simulink model "ofdm_txrx_supermimo_coded"  (Simulink 7.7)
settings: Solver=VariableStepDiscrete  SolverMode=SingleTasking  StartTime=0.0  StopTime=2*simOnly_simLength  RelTol=1e-3
PostLoadFcn:
  | ofdm_rx_supermimo_init
InitFcn:
  | ofdm_rx_supermimo_init
StartFcn:
  | ofdm_rx_supermimo_init
values from neighbouring files (symbols this model uses):
  ActionID_AFTransmit = 31    [ofdm_tx_supermimo_init.m]
  ActionID_Disabled = 0    [ofdm_tx_supermimo_init.m]
  ActionID_SetFlagA = 62    [ofdm_tx_supermimo_init.m]
  ActionID_SetFlagB = 61    [ofdm_tx_supermimo_init.m]
  BER_RAM_init_size = 2048    [ofdm_tx_supermimo_init.m]
  channel_h = [1 0; 1 0] * 0.3    [ofdm_tx_supermimo_init.m]
  CPLength = 16    [ofdm_tx_supermimo_init.m]
  longCorr_coef_i = [-4*real(LTS) 0]    [ofdm_rx_supermimo_init.m]
  longCorr_coef_q = [4*imag(LTS) 0]    [ofdm_rx_supermimo_init.m]
  max_CP_length = 16    [ofdm_tx_supermimo_init.m]
  max_num_baseRateSymbols = 31    [ofdm_tx_supermimo_init.m]
  max_num_subcarriers = 64    [ofdm_tx_supermimo_init.m]
  max_num_trainingSymbols = 15    [ofdm_tx_supermimo_init.m]
  max_numBytes = 16384    [ofdm_tx_supermimo_init.m]
  max_OFDM_symbols = 1023    [ofdm_tx_supermimo_init.m]
  modConstellation_qam16 = .75*[1 3 -1 -3]./3    [ofdm_tx_supermimo_init.m]
  modConstellation_qam64 = 0.875*[3 1 5 7 -3 -1 -5 -7]./7    [ofdm_tx_supermimo_init.m]
  modConstellation_qpsk = [1 -1]./sqrt(2)    [ofdm_tx_supermimo_init.m]
  numBaseRateSymbols = numBaseRateSymbols *2    [ofdm_tx_supermimo_init.m]
  numSubcarriers = 64    [ofdm_tx_supermimo_init.m]
  numTrainingSymbols = 2    [ofdm_tx_supermimo_init.m]
  pktTiming_count_payloadStart = pktTiming_count_lsStart + pktTiming_ls_num*pktTiming_ls_length    [ofdm_rx_supermimo_init.m]
  preamble = preamble_scale*[shortsyms_10 longsyms_2]    [ofdm_tx_supermimo_init.m]
  preamble_ROM = preamble_I_ROM + preamble_Q_ROM*2^16    [ofdm_tx_supermimo_init.m]
  RAM_init_size = max_numBytes    (= 16384)    [ofdm_tx_supermimo_init.m]
  RxDataScrambling_Seq = [40 198 78 63 82 173 102 245 48 111 172 115 147 230 216 93 72 65 62 2 205 242 122 90 128 83 105 97 73 10 5 252]    [ofdm_tx_supermimo_init.m]
  shortSymbol_time = shortSymbol_time(1:16).'    [ofdm_tx_supermimo_init.m]
  simOnly_numSamples = length(preamble)+( (numSubcarriers+CPLength)*(numTrainingSymbols + numBaseRateSymbols + numFullRateSymbols/2) )    [ofdm_tx_supermimo_init.m]
  train = [train train]    [ofdm_tx_supermimo_init.m]
  TxDataScrambling_Seq = [40 198 78 63 82 173 102 245 48 111 172 115 147 230 216 93 72 65 62 2 205 242 122 90 128 83 105 97 73 10 5 252]    [ofdm_tx_supermimo_init.m]
undefined symbols (not defined in the file or in its folder): AntA, AntB, BaseRate, Down, gcbh, numBuffs, numBytes, Up, xlBlockLoadCallback, xlGetNormalizedPeriod, xlipgetpartsetting
libraries: xbsIndex_r4 x5430
(part 5 of 49)

== system ofdm_txrx_supermimo_coded/Memory-mapped Registers/TxRx Registers ==
  16LSB+8 : xbsIndex_r4/Slice  nbits=16  boolean_output=off  mode=Lower Bit Location + Width  bit1=0  base1=MSB of Input  bit0=8  base0=LSB of Input  dbl_ovrd=off  block_type=slice
      settings: infoedit="Extracts a given range of bits from each input sample and presents it at the output.  The output type is ordinarily unsigned with binary point at zero, but can be Boolean when the slice is one bit wide.<P><P>Hardware notes: In hardware this block costs nothing."  block_version=VER_STRING_GOES_HERE
  16LSB1 : xbsIndex_r4/Slice  nbits=ceil(log2(max_num_trainingSymbols))  boolean_output=off  mode=Lower Bit Location + Width  bit1=0  base1=MSB of Input  bit0=0  base0=LSB of Input  dbl_ovrd=off  block_type=slice
      settings: infoedit="Extracts a given range of bits from each input sample and presents it at the output.  The output type is ordinarily unsigned with binary point at zero, but can be Boolean when the slice is one bit wide.<P><P>Hardware notes: In hardware this block costs nothing."  block_version=VER_STRING_GOES_HERE
  16MSB1 : xbsIndex_r4/Slice  nbits=ceil(log2(max_num_baseRateSymbols))  boolean_output=off  mode=Lower Bit Location + Width  bit1=0  base1=MSB of Input  bit0=16  base0=LSB of Input  dbl_ovrd=off  block_type=slice
      settings: infoedit="Extracts a given range of bits from each input sample and presents it at the output.  The output type is ordinarily unsigned with binary point at zero, but can be Boolean when the slice is one bit wide.<P><P>Hardware notes: In hardware this block costs nothing."  block_version=VER_STRING_GOES_HERE
  6LSB+6 : xbsIndex_r4/Slice  nbits=6  boolean_output=off  mode=Lower Bit Location + Width  bit1=0  base1=MSB of Input  bit0=6  base0=LSB of Input  dbl_ovrd=off  block_type=slice
      settings: infoedit="Extracts a given range of bits from each input sample and presents it at the output.  The output type is ordinarily unsigned with binary point at zero, but can be Boolean when the slice is one bit wide.<P><P>Hardware notes: In hardware this block costs nothing."  block_version=VER_STRING_GOES_HERE
  6LSB1 : xbsIndex_r4/Slice  nbits=6  boolean_output=off  mode=Lower Bit Location + Width  bit1=0  base1=MSB of Input  bit0=0  base0=LSB of Input  dbl_ovrd=off  block_type=slice
      settings: infoedit="Extracts a given range of bits from each input sample and presents it at the output.  The output type is ordinarily unsigned with binary point at zero, but can be Boolean when the slice is one bit wide.<P><P>Hardware notes: In hardware this block costs nothing."  block_version=VER_STRING_GOES_HERE
  8LSB : xbsIndex_r4/Slice  nbits=8  boolean_output=off  mode=Lower Bit Location + Width  bit1=0  base1=MSB of Input  bit0=0  base0=LSB of Input  dbl_ovrd=off  block_type=slice
      settings: infoedit="Extracts a given range of bits from each input sample and presents it at the output.  The output type is ordinarily unsigned with binary point at zero, but can be Boolean when the slice is one bit wide.<P><P>Hardware notes: In hardware this block costs nothing."  block_version=VER_STRING_GOES_HERE
  AddSub1 : xbsIndex_r4/AddSub  mode=Addition  use_carryin=off  use_carryout=off  en=off  latency=0  precision=User Defined  arith_type=Unsigned  n_bits=1 + ceil(log2(max_num_trainingSymbols + max_num_baseRateSymbols))  bin_pt=0  quantization=Truncate  overflow=Wrap  dbl_ovrd=off  use_behavioral_HDL=on  hw_selection=Fabric  pipelined=off  use_rpm=on  block_type=addsub
      settings: xl_area=[0, 0, 0, 0, 0, 0, 0]  block_version=VER_STRING_GOES_HERE
  Down Sample{1,4,7,11,25} (5 alike) : xbsIndex_r4/Down Sample  sample_ratio=2  sample_phase="Last Value of Frame  (most efficient)"  en=off  latency=1  dbl_ovrd=off  block_type=dsamp
      settings: block_version=VER_STRING_GOES_HERE
  From Register2 : xbsIndex_r4/From Register  shared_memory_name='TxRx_FFT_Scaling'  init=TxRx_FFTScaling  period=1  ownership=Locally owned and initialized  gui_display_data_type=Fixed-point  arith_type=Unsigned  n_bits=12  bin_pt=0  preci_type=Single  dbl_ovrd=off  block_type=fromreg
      settings: AttributesFormatString=<< %<shared_memory_name> >>  infoedit="Register block that reads data to a shared memory register.  Delay of one sample period."
  From Register3 : xbsIndex_r4/From Register  shared_memory_name='TxRx_Interrupt_PktBuf_Ctrl'  init=reg_Interrupt_PktBuf_Control  period=1  ownership=Locally owned and initialized  gui_display_data_type=Fixed-point  arith_type=Unsigned  n_bits=32  bin_pt=0  preci_type=Single  dbl_ovrd=off  block_type=fromreg
      settings: AttributesFormatString=<< %<shared_memory_name> >>  infoedit="Register block that reads data to a shared memory register.  Delay of one sample period."
  From Register4 : xbsIndex_r4/From Register  shared_memory_name='TxRx_Pilots_Index'  init=pilot_indicies  period=1  ownership=Locally owned and initialized  gui_display_data_type=Fixed-point  arith_type=Unsigned  n_bits=32  bin_pt=0  preci_type=Single  dbl_ovrd=off  block_type=fromreg
      settings: AttributesFormatString=<< %<shared_memory_name> >>  infoedit="Register block that reads data to a shared memory register.  Delay of one sample period."
  From Register5 : xbsIndex_r4/From Register  shared_memory_name='TxRx_Pilots_Values'  init=pilotValues  period=1  ownership=Locally owned and initialized  gui_display_data_type=Fixed-point  arith_type=Unsigned  n_bits=32  bin_pt=0  preci_type=Single  dbl_ovrd=off  block_type=fromreg
      settings: AttributesFormatString=<< %<shared_memory_name> >>  infoedit="Register block that reads data to a shared memory register.  Delay of one sample period."
  From Register6 : xbsIndex_r4/From Register  shared_memory_name='Rx_OFDM_SymbolCounts'  init=(2^16 * numBaseRateSymbols) + numTrainingSymbols  period=1  ownership=Locally owned and initialized  gui_display_data_type=Fixed-point  arith_type=Unsigned  n_bits=32  bin_pt=0  preci_type=Single  dbl_ovrd=off  block_type=fromreg
      settings: AttributesFormatString=<< %<shared_memory_name> >>  infoedit="Register block that reads data to a shared memory register.  Delay of one sample period."
  From Register7 : xbsIndex_r4/From Register  shared_memory_name='Tx_OFDM_SymCounts'  init=txReg_symbolCounts  period=1  ownership=Locally owned and initialized  gui_display_data_type=Fixed-point  arith_type=Unsigned  n_bits=32  bin_pt=0  preci_type=Single  dbl_ovrd=off  block_type=fromreg
      settings: AttributesFormatString=<< %<shared_memory_name> >>  infoedit="Register block that reads data to a shared memory register.  Delay of one sample period."
  From Register8 : xbsIndex_r4/From Register  shared_memory_name='FEC_Config'  init=FEC_Config  period=1  ownership=Locally owned and initialized  gui_display_data_type=Fixed-point  arith_type=Unsigned  n_bits=32  bin_pt=0  preci_type=Single  dbl_ovrd=off  block_type=fromreg
      settings: AttributesFormatString=<< %<shared_memory_name> >>  infoedit="Register block that reads data to a shared memory register.  Delay of one sample period."
  InterruptControl : Subsystem
  LSB : xbsIndex_r4/Slice  nbits=1  boolean_output=off  mode=Lower Bit Location + Width  bit1=0  base1=MSB of Input  bit0=0  base0=LSB of Input  dbl_ovrd=off  block_type=slice
      settings: infoedit="Extracts a given range of bits from each input sample and presents it at the output.  The output type is ordinarily unsigned with binary point at zero, but can be Boolean when the slice is one bit wide.<P><P>Hardware notes: In hardware this block costs nothing."  block_version=VER_STRING_GOES_HERE
  tag scope: Coding_En=global, FEC_Reg=global, Rx_FFTScaling=global, TxReg_OFDM_SymCounts=global, Tx_FFTScaling=global, regRx_numBaseRateSymbols=global, regRx_numTrainPlusBaseRateSyms=global, regRx_numTrainingSymbols=global, regTxRx_PilotToneIndex=global, regTxRx_PktBuffOffsets=global, regTx_PilotToneValues=global
  wires:
  From Register3/1 -> Down Sample7/1, 16LSB+8/1
  Down Sample7/1 -> 8LSB/1
  From Register2/1 -> Down Sample25/1
  Down Sample25/1 -> 6LSB1/1, 6LSB+6/1
  6LSB1/1 -> [Tx_FFTScaling]
  6LSB+6/1 -> [Rx_FFTScaling]
  16LSB+8/1 -> [regTxRx_PktBuffOffsets]
  8LSB/1 -> InterruptControl/1(Reg_8b)
  From Register4/1 -> Down Sample4/1
  Down Sample4/1 -> [regTxRx_PilotToneIndex]
  From Register5/1 -> [regTx_PilotToneValues]
  From Register7/1 -> [TxReg_OFDM_SymCounts]
  AddSub1/1 -> [regRx_numTrainPlusBaseRateSyms]
  16LSB1/1 -> [regRx_numTrainingSymbols], AddSub1/1
  16MSB1/1 -> [regRx_numBaseRateSymbols], AddSub1/2
  From Register6/1 -> Down Sample11/1
  Down Sample11/1 -> 16MSB1/1, 16LSB1/1
  From Register8/1 -> LSB/1, [FEC_Reg]
  LSB/1 -> Down Sample1/1
  Down Sample1/1 -> [Coding_En]

== system ofdm_txrx_supermimo_coded/OFDM Rx MIMO/ADC Inputs & Antenna Selection ==
  note: Extra latency to cover delay through
      | MAX2829 amps -> ADC -> FPGA
  PktDetReset : Inport
  "A I " : Inport  Port=2
  "A Q " : Inport  Port=3
  "B I " : Inport  Port=4
  "B Q " : Inport  Port=5
  Antenna Selection : Subsystem
  Constant4 : Constant
  Constant5 : Constant
  Convert : xbsIndex_r4/Convert  gui_display_data_type=Fixed-point  arith_type=Boolean  n_bits=16  bin_pt=14  float_type=Single  exp_bits=8  fraction_bits=24  quantization=Truncate  overflow=Wrap  en=off  latency=0  dbl_ovrd=off  pipeline=off  block_type=convert
      settings: block_version=10.1.3
  Convert1 : xbsIndex_r4/Convert  gui_display_data_type=Fixed-point  arith_type=Boolean  n_bits=16  bin_pt=14  float_type=Single  exp_bits=8  fraction_bits=24  quantization=Truncate  overflow=Wrap  en=off  latency=0  dbl_ovrd=off  pipeline=off  block_type=convert
      settings: block_version=10.1.3
  Down Sample{,1..3} (4 alike) : xbsIndex_r4/Down Sample  sample_ratio=4  sample_phase="Last Value of Frame  (most efficient)"  en=off  latency=1  dbl_ovrd=off  block_type=dsamp
      settings: block_version=8.2.02
  Down Sample6 : xbsIndex_r4/Down Sample  sample_ratio=4  sample_phase="Last Value of Frame  (most efficient)"  en=off  latency=4  dbl_ovrd=off  block_type=dsamp
      settings: block_version=8.2.02
  Down Sample9 : xbsIndex_r4/Down Sample  sample_ratio=4  sample_phase="Last Value of Frame  (most efficient)"  en=off  latency=4  dbl_ovrd=off  block_type=dsamp
      settings: block_version=8.2.02
  Inverter : xbsIndex_r4/Inverter  en=off  latency=0  dbl_ovrd=off  block_type=inv
  Inverter1 : xbsIndex_r4/Inverter  en=off  latency=0  dbl_ovrd=off  block_type=inv
  Inverter2 : xbsIndex_r4/Inverter  en=off  latency=0  dbl_ovrd=off  block_type=inv
  Logical1 : xbsIndex_r4/Logical  logical_function=AND  inputs=2  en=off  latency=0  precision=Full  arith_type=Unsigned  n_bits=8  bin_pt=2  align_bp=on  dbl_ovrd=off  block_type=logical
      settings: xl_area=[1 0 0 1 0 0 0]  block_version=VER_STRING_GOES_HERE
  Logical2 : xbsIndex_r4/Logical  logical_function=OR  inputs=2  en=off  latency=0  precision=Full  arith_type=Unsigned  n_bits=8  bin_pt=2  align_bp=on  dbl_ovrd=off  block_type=logical
      settings: xl_area=[1 0 0 1 0 0 0]  block_version=VER_STRING_GOES_HERE
  Logical3 : xbsIndex_r4/Logical  logical_function=AND  inputs=2  en=off  latency=0  precision=Full  arith_type=Unsigned  n_bits=8  bin_pt=2  align_bp=on  dbl_ovrd=off  block_type=logical
      settings: xl_area=[1 0 0 1 0 0 0]  block_version=VER_STRING_GOES_HERE
  Register{,1..3} (4 alike) : xbsIndex_r4/Register  init=0  rst=on  en=off  dbl_ovrd=off  block_type=register
  Rx_AntA_ADCI : xbsIndex_r4/Gateway In  gui_display_data_type=Fixed-point  arith_type="Signed  (2's comp)"  n_bits=14  bin_pt=13  preci_type=Single  exp_width=8  frac_width=24  quantization="Round  (unbiased: +/- Inf)"  overflow=Saturate  period=1  dbl_ovrd=off  timing_constraint=None  locs_specified=off  LOCs={}  inherit_from_input=off  UseAsADC=off  ADCChannel='1'  hdl_port=on  block_type=gatewayin
      settings: infoedit="Gateway in block.  Converts inputs of type Simulink integer, double and fixed point to  Xilinx fixed point type.<P><P>Hardware notes:  In hardware these blocks become top level input ports."  block_version=VER_STRING_GOES_HERE
  Rx_AntA_ADCQ : xbsIndex_r4/Gateway In  gui_display_data_type=Fixed-point  arith_type="Signed  (2's comp)"  n_bits=14  bin_pt=13  preci_type=Single  exp_width=8  frac_width=24  quantization="Round  (unbiased: +/- Inf)"  overflow=Saturate  period=1  dbl_ovrd=off  timing_constraint=None  locs_specified=off  LOCs={}  inherit_from_input=off  UseAsADC=off  ADCChannel='1'  hdl_port=on  block_type=gatewayin
      settings: infoedit="Gateway in block.  Converts inputs of type Simulink integer, double and fixed point to  Xilinx fixed point type.<P><P>Hardware notes:  In hardware these blocks become top level input ports."  block_version=VER_STRING_GOES_HERE
  Rx_AntA_AGC_Done : xbsIndex_r4/Gateway In  gui_display_data_type=Fixed-point  arith_type=Unsigned  n_bits=1  bin_pt=0  preci_type=Single  exp_width=8  frac_width=24  quantization="Round  (unbiased: +/- Inf)"  overflow=Saturate  period=1  dbl_ovrd=off  timing_constraint=None  locs_specified=off  LOCs={}  inherit_from_input=off  UseAsADC=off  ADCChannel='1'  hdl_port=on  block_type=gatewayin
      settings: infoedit="Gateway in block.  Converts inputs of type Simulink integer, double and fixed point to  Xilinx fixed point type.<P><P>Hardware notes:  In hardware these blocks become top level input ports."  block_version=VER_STRING_GOES_HERE
  Rx_AntB_ADCI : xbsIndex_r4/Gateway In  gui_display_data_type=Fixed-point  arith_type="Signed  (2's comp)"  n_bits=14  bin_pt=13  preci_type=Single  exp_width=8  frac_width=24  quantization="Round  (unbiased: +/- Inf)"  overflow=Saturate  period=1  dbl_ovrd=off  timing_constraint=None  locs_specified=off  LOCs={}  inherit_from_input=off  UseAsADC=off  ADCChannel='1'  hdl_port=on  block_type=gatewayin
      settings: infoedit="Gateway in block.  Converts inputs of type Simulink integer, double and fixed point to  Xilinx fixed point type.<P><P>Hardware notes:  In hardware these blocks become top level input ports."  block_version=VER_STRING_GOES_HERE
  Rx_AntB_ADCQ : xbsIndex_r4/Gateway In  gui_display_data_type=Fixed-point  arith_type="Signed  (2's comp)"  n_bits=14  bin_pt=13  preci_type=Single  exp_width=8  frac_width=24  quantization="Round  (unbiased: +/- Inf)"  overflow=Saturate  period=1  dbl_ovrd=off  timing_constraint=None  locs_specified=off  LOCs={}  inherit_from_input=off  UseAsADC=off  ADCChannel='1'  hdl_port=on  block_type=gatewayin
      settings: infoedit="Gateway in block.  Converts inputs of type Simulink integer, double and fixed point to  Xilinx fixed point type.<P><P>Hardware notes:  In hardware these blocks become top level input ports."  block_version=VER_STRING_GOES_HERE
  Rx_AntB_AGC_Done : xbsIndex_r4/Gateway In  gui_display_data_type=Fixed-point  arith_type=Unsigned  n_bits=1  bin_pt=0  preci_type=Single  exp_width=8  frac_width=24  quantization="Round  (unbiased: +/- Inf)"  overflow=Saturate  period=1  dbl_ovrd=off  timing_constraint=None  locs_specified=off  LOCs={}  inherit_from_input=off  UseAsADC=off  ADCChannel='1'  hdl_port=on  block_type=gatewayin
      settings: infoedit="Gateway in block.  Converts inputs of type Simulink integer, double and fixed point to  Xilinx fixed point type.<P><P>Hardware notes:  In hardware these blocks become top level input ports."  block_version=VER_STRING_GOES_HERE
  A I : Outport
  A Q : Outport  Port=2
  B I : Outport  Port=3
  B Q : Outport  Port=4
  tag scope: AntA_ADC_I_Filt=global, AntA_ADC_Q_Filt=global, AntA_AGC_Done=global, AntB_AGC_Done=global
  wires:
  "B Q "/1 -> Rx_AntB_ADCQ/1
  "B I "/1 -> Rx_AntB_ADCI/1
  "A Q "/1 -> Rx_AntA_ADCQ/1
  "A I "/1 -> Rx_AntA_ADCI/1
  Down Sample/1 -> Antenna Selection/1(AntA_I)
  Down Sample1/1 -> Antenna Selection/2(AntA_Q)
  Down Sample2/1 -> Antenna Selection/3(AntB_I)
  Down Sample3/1 -> Antenna Selection/4(AntB_Q)
  Antenna Selection/1(A I) -> [AntA_ADC_I_Filt], Register/1
  Antenna Selection/2(A Q) -> [AntA_ADC_Q_Filt], Register1/1
  PktDetReset/1 -> Antenna Selection/5(PktDetReset)
  Constant5/1 -> Rx_AntB_AGC_Done/1
  Rx_AntB_AGC_Done/1 -> Down Sample9/1
  Down Sample9/1 -> [AntB_AGC_Done], Convert1/1
  Constant4/1 -> Rx_AntA_AGC_Done/1
  Rx_AntA_AGC_Done/1 -> Down Sample6/1
  Down Sample6/1 -> [AntA_AGC_Done], Convert/1
  Rx_AntA_ADCI/1 -> Down Sample/1
  Rx_AntA_ADCQ/1 -> Down Sample1/1
  Rx_AntB_ADCI/1 -> Down Sample2/1
  Rx_AntB_ADCQ/1 -> Down Sample3/1
  Register/1 -> A I/1
  Register1/1 -> A Q/1
  Register2/1 -> B I/1
  Register3/1 -> B Q/1
  Antenna Selection/4(B Q) -> Register3/1
  Antenna Selection/3(B I) -> Register2/1
  Inverter1/1 -> Register2/2, Register3/2, Logical3/2
  Convert/1 -> Inverter/1
  Convert1/1 -> Inverter1/1
  Inverter/1 -> Logical1/2
  Inverter2/1 -> Logical1/1
  Antenna Selection/5(A_swapped) -> Inverter2/1, Logical3/1
  Logical1/1 -> Logical2/1
  Logical3/1 -> Logical2/2
  Logical2/1 -> Register1/2, Register/2

== system ofdm_txrx_supermimo_coded/OFDM Rx MIMO/AF Buffers ==
  PktDone : Inport
  Payload : Inport  Port=2
  AntA_I : Inport  Port=3
  AntA_Q : Inport  Port=4
  AF Buffers : Subsystem
  tag scope: AF_OutputSamples_I=global, AF_OutputSamples_Q=global, AF_PreambleIndex=global, AF_TxDone=global
  wires:
  AntA_I/1 -> AF Buffers/1(AntA_I)
  AntA_Q/1 -> AF Buffers/2(AntA_Q)
  Payload/1 -> AF Buffers/3(Payload)
  PktDone/1 -> AF Buffers/4(PktDone)
  AF Buffers/1(I) -> [AF_OutputSamples_I]
  AF Buffers/2(Q) -> [AF_OutputSamples_Q]
  AF Buffers/3(AF Pream Index) -> [AF_PreambleIndex]
  AF Buffers/4(AFTx Done) -> [AF_TxDone]

== system ofdm_txrx_supermimo_coded/OFDM Rx MIMO/Coarse Freq Correction ==
  note: Potential for overflow pre-AGC
      | (but not post? I think it's ok)
      | |
      | V
  Reset : Inport
  PktDet : Inport  Port=2
  AntA I : Inport  Port=3
  AntA Q : Inport  Port=4
  AntB I : Inport  Port=5
  AntB Q : Inport  Port=6
  A*B : Subsystem
  A*B1 : Subsystem
  Bus Creator1 : BusCreator  Inputs=2
  CFO : Scope  NumInputPorts=5
      settings: TimeRange=14608  YMin=0.000246735~0.95~-0.3~-1~-1  YMax=0.000272707~1.05~1~1~1  SaveName=ScopeData26
  Coarse CFO Calc : Subsystem
  Complex to Magnitude-Angle : ComplexToMagnitudeAngle
  Complex to Real-Imag : ComplexToRealImag
  Convert{,1..3} (4 alike) : xbsIndex_r4/Convert  gui_display_data_type=Fixed-point  arith_type="Signed  (2's comp)"  n_bits=16  bin_pt=15  float_type=Single  exp_bits=8  fraction_bits=24  quantization=Truncate  overflow=Wrap  en=off  latency=0  dbl_ovrd=off  pipeline=off  block_type=convert
      settings: xl_area=[0 0 0 0 0 0 0]  block_version=VER_STRING_GOES_HERE
  DDS : Subsystem
  Gateway Out{,1..3} (4 alike) : xbsIndex_r4/Gateway Out  inherit_from_input=off  hdl_port=off  timing_constraint=None  locs_specified=off  LOCs={}  UseAsDAC=off  DACChannel='1'  block_type=gatewayout
      settings: infoedit="Gateway out block.  Converts Xilinx fixed point inputs into ouputs of type Simulink integer, double, or fixed point.<P><P>Hardware notes:  In hardware these blocks become top level output ports or are discarded, depending on how they are configured."  xl_area=[0 0 0 0 0 0 0]  block_version=VER_STRING_GOES_HERE
  Mux2 : Mux  Inputs=2
  PreSpin CFO Sel : Subsystem
  Product : Product  RndMeth=Zero
  Real-Imag to Complex : RealImagToComplex
  "AntA I " : Outport
  "AntA Q " : Outport  Port=2
  "AntB I " : Outport  Port=3
  "AntB Q " : Outport  Port=4
  tag scope: CFO_PhaseInc=global
  wires:
  A*B/1(Re) -> Convert/1
  A*B/2(Im1) -> Convert1/1
  A*B1/1(Re) -> Convert2/1
  A*B1/2(Im1) -> Convert3/1
  DDS/1(Q) -> Gateway Out/1, A*B1/2(Im[B]), A*B/3(Im[B])
  DDS/2(I) -> Gateway Out1/1, A*B1/1(Re[B]), A*B/4(Re[B])
  Gateway Out1/1 -> Mux2/1, Real-Imag to Complex/1
  Gateway Out/1 -> Mux2/2, Real-Imag to Complex/2
  Mux2/1 -> CFO/3
  Bus Creator1/1 -> CFO/4
  [freqOffset] -> Complex to Real-Imag/1, Product/2
  Complex to Real-Imag/1 -> Bus Creator1/1
  Complex to Real-Imag/2 -> Bus Creator1/2
  Gateway Out2/1 -> CFO/2
  Gateway Out3/1 -> CFO/1
  AntB I/1 -> A*B1/3(Re[A])
  AntB Q/1 -> A*B1/4(Im[A])
  AntA I/1 -> A*B/1(Re[A]), Coarse CFO Calc/1(I In)
  AntA Q/1 -> A*B/2(Im[A]), Coarse CFO Calc/2(Q In)
  Convert/1 -> "AntA I "/1
  Convert1/1 -> "AntA Q "/1
  Real-Imag to Complex/1 -> Product/1
  Product/1 -> Complex to Magnitude-Angle/1
  Complex to Magnitude-Angle/2 -> CFO/5
  Convert2/1 -> "AntB I "/1
  Convert3/1 -> "AntB Q "/1
  Reset/1 -> Coarse CFO Calc/4(Reset), DDS/3(Rst), PreSpin CFO Sel/1(Rst)
  PktDet/1 -> Gateway Out2/1, Coarse CFO Calc/3(RxPkt Active)
  Coarse CFO Calc/1(Phase Inc) -> Gateway Out3/1, [CFO_PhaseInc], DDS/2(Rx_CoarseEst)
  PreSpin CFO Sel/1(Tx_PhaseInc) -> DDS/1(Tx_PhaseInc)

== system ofdm_txrx_supermimo_coded/OFDM Rx MIMO/Equalizer & Packetizer ==
  Payload : Inport
  Y_I : Inport  Port=2
  Y_Q : Inport  Port=3
  H-I : Inport  Port=4
  H-Q : Inport  Port=5
  SubCar Index : Inport  Port=6
  Sym Index : Inport  Port=7
  Vin : Inport  Port=8
  Phases I : Inport  Port=9
  Phases Q : Inport  Port=10
  Convert4 : xbsIndex_r4/Convert  gui_display_data_type=Fixed-point  arith_type="Signed  (2's comp)"  n_bits=14  bin_pt=13  float_type=Single  exp_bits=8  fraction_bits=24  quantization=Truncate  overflow=Wrap  en=off  latency=0  dbl_ovrd=off  pipeline=off  block_type=convert
      settings: xl_area=[0, 0, 0, 0, 0, 0, 0]  block_version=VER_STRING_GOES_HERE
  Convert5 : xbsIndex_r4/Convert  gui_display_data_type=Fixed-point  arith_type="Signed  (2's comp)"  n_bits=14  bin_pt=13  float_type=Single  exp_bits=8  fraction_bits=24  quantization=Truncate  overflow=Wrap  en=off  latency=0  dbl_ovrd=off  pipeline=off  block_type=convert
      settings: xl_area=[0, 0, 0, 0, 0, 0, 0]  block_version=VER_STRING_GOES_HERE
  Eq : Scope  NumInputPorts=7
      settings: YMin=-0.5~-0.5~0~0~0~0~-1  YMax=0.5~0.5~70~1~22.5~1~1  SaveName=ScopeData14
  Equalizer : Subsystem
  Packet_Constructor : Subsystem
  Real-Imag to Complex : RealImagToComplex
  Rx_debug_EQ_I : xbsIndex_r4/Gateway Out  inherit_from_input=off  hdl_port=on  timing_constraint=None  locs_specified=off  LOCs={}  UseAsDAC=off  DACChannel='1'  block_type=gatewayout
      settings: infoedit="Gateway out block.  Converts Xilinx fixed point inputs into ouputs of type Simulink integer, double, or fixed point.<P><P>Hardware notes:  In hardware these blocks become top level output ports or are discarded, depending on how they are configured."  xl_area=[0 0 0 0 1 0 0]  block_version=VER_STRING_GOES_HERE
  Rx_debug_EQ_Q : xbsIndex_r4/Gateway Out  inherit_from_input=off  hdl_port=on  timing_constraint=None  locs_specified=off  LOCs={}  UseAsDAC=off  DACChannel='1'  block_type=gatewayout
      settings: infoedit="Gateway out block.  Converts Xilinx fixed point inputs into ouputs of type Simulink integer, double, or fixed point.<P><P>Hardware notes:  In hardware these blocks become top level output ports or are discarded, depending on how they are configured."  xl_area=[0 0 0 0 1 0 0]  block_version=VER_STRING_GOES_HERE
  Scaling : Subsystem
  Up Sample1 : xbsIndex_r4/Up Sample  sample_ratio=2  copy_samples=on  en=off  latency=0  dbl_ovrd=off  block_type=usamp
      settings: infoedit="Up samples input data.  Inserted values  can be zeros or copies of the most recent input sample.<P><P>Hardware notes: No hardware is needed if inserted values are copies of the input sample; otherwise, a mux and single bit flip-flop are used."  block_version=8.2.02
  Up Sample2 : xbsIndex_r4/Up Sample  sample_ratio=2  copy_samples=on  en=off  latency=0  dbl_ovrd=off  block_type=usamp
      settings: infoedit="Up samples input data.  Inserted values  can be zeros or copies of the most recent input sample.<P><P>Hardware notes: No hardware is needed if inserted values are copies of the input sample; otherwise, a mux and single bit flip-flop are used."  block_version=8.2.02
  Zero-Order Hold : ZeroOrderHold
  Zero-Order Hold1 : ZeroOrderHold
  done{1..6} (6 alike) : xbsIndex_r4/Gateway Out  inherit_from_input=off  hdl_port=off  timing_constraint=None  locs_specified=off  LOCs={}  UseAsDAC=off  DACChannel='1'  block_type=gatewayout
      settings: infoedit="Gateway out block.  Converts Xilinx fixed point inputs into ouputs of type Simulink integer, double, or fixed point.<P><P>Hardware notes:  In hardware these blocks become top level output ports or are discarded, depending on how they are configured."  xl_area=[0 0 0 0 1 0 0]  block_version=VER_STRING_GOES_HERE
  posedge : Subsystem
  done : Outport
  wires:
  SubCar Index/1 -> Equalizer/9(SubCar Index)
  Sym Index/1 -> Equalizer/8(Sym Index)
  Scaling/1(I) -> Packet_Constructor/1(I), Convert4/1
  Scaling/2(Q) -> Packet_Constructor/2(Q), Convert5/1
  Scaling/3(" Xk_index") -> Packet_Constructor/3(Xk_index)
  Scaling/4(Vout) -> Packet_Constructor/4(Vin)
  Scaling/5(" Sym Index") -> Packet_Constructor/5(Sym Index)
  Packet_Constructor/1(done) -> done/1
  H-I/1 -> Equalizer/5(H-I)
  H-Q/1 -> Equalizer/6(H-Q)
  Vin/1 -> Equalizer/7(Vin)
  Y_I/1 -> Equalizer/3(Y_I)
  Y_Q/1 -> Equalizer/4(Y_Q)
  Payload/1 -> posedge/1(D), done6/1
  posedge/1(Q) -> Packet_Constructor/6(Start)
  Equalizer/1(I-eq) -> Scaling/1(Eq-I), done4/1
  Equalizer/2(Q-eq) -> Scaling/2(Eq-Q), done5/1
  Equalizer/3(" Xk_index") -> Scaling/3(Xk_index), done1/1
  Equalizer/4(Vout) -> Scaling/4(Vin), done2/1
  Equalizer/5(" Sym Index") -> Scaling/5(Sym Index), done3/1
  Up Sample2/1 -> Rx_debug_EQ_Q/1
  Up Sample1/1 -> Rx_debug_EQ_I/1
  Convert5/1 -> Up Sample2/1
  Convert4/1 -> Up Sample1/1
  Phases I/1 -> Equalizer/1(Phases I)
  Phases Q/1 -> Equalizer/2(Phases Q)
  Rx_debug_EQ_I/1 -> Zero-Order Hold/1
  Rx_debug_EQ_Q/1 -> Zero-Order Hold1/1
  Real-Imag to Complex/1 -> ?
  Zero-Order Hold/1 -> Real-Imag to Complex/1
  Zero-Order Hold1/1 -> Real-Imag to Complex/2
  done4/1 -> Eq/1
  done5/1 -> Eq/2
  done1/1 -> Eq/3
  done2/1 -> Eq/4
  done3/1 -> Eq/5
  done6/1 -> Eq/6

== system ofdm_txrx_supermimo_coded/OFDM Rx MIMO/FFT & Chan Est ==
  note: Payload->WE latency was 1 - why?
  Payload : Inport
  AntA I : Inport  Port=2
  AntA Q : Inport  Port=3
  AntB I : Inport  Port=4
  AntB Q : Inport  Port=5
  Addr Gen1 : Subsystem
  Addr_Generator : Subsystem
  Assert : xbsIndex_r4/Assert  assert_type=off  type_source=Explicitly  gui_display_data_type=Fixed-point  arith_type="Signed  (2's comp)"  n_bits=16  bin_pt=14  preci_type=Single  exp_width=8  frac_width=24  assert_rate=on  rate_source=Explicitly  period=1  output_port=on  block_type=assert
      settings: infoedit=Asserts a user-defined sample rate and/or type on a signal.<P><P>Hardware notes: In hardware this block costs nothing.  block_version=VER_STRING_GOES_HERE
  Channel Estimation & Pilot Processing : Subsystem
  Delay{1..4} (4 alike) : xbsIndex_r4/Delay  rst=off  infoeditControl=Selection of Reset will increase slice count due to use of real FFs and instead of SRLs  en=off  latency=1  dbl_ovrd=off  reg_retiming=off  block_type=delay
      settings: infoedit=Hardware notes: A delay line is a chain, each link of which is an SRL16 followed by a flip-flop. If register retiming is enabled, the delay line is a chain of flip-flops.  block_version=VER_STRING_GOES_HERE
  Down Sample : xbsIndex_r4/Down Sample  sample_ratio=2  sample_phase=First Value of Frame  en=off  latency=0  dbl_ovrd=off  block_type=dsamp
      settings: block_version=VER_STRING_GOES_HERE
  FFT : Subsystem
  Input Buffers : Subsystem
  Inverter2 : xbsIndex_r4/Inverter  en=off  latency=0  dbl_ovrd=off  block_type=inv
      settings: block_version=VER_STRING_GOES_HERE
  Output Buffers : Subsystem
  Symbol Counter : Subsystem
  Y_I : Outport
  Y_Q : Outport  Port=2
  H-I : Outport  Port=3
  H-Q : Outport  Port=4
  SubCar Index : Outport  Port=5
  Sym Index : Outport  Port=6
  Vout : Outport  Port=7
  Phase I : Outport  Port=8
  Phase Q : Outport  Port=9
  tag scope: FFT_I=global
  wires:
  Delay1/1 -> Input Buffers/5(We)
  FFT/5(Done) -> Symbol Counter/1(FFT Done), Addr Gen1/1(FFT Done)
  Addr_Generator/3(Ant Sel) -> Input Buffers/8(Ant Sel)
  Assert/1 -> Addr_Generator/2(FFT Xn_index)
  AntB Q/1 -> Input Buffers/4(AntB Q)
  AntB I/1 -> Input Buffers/3(AntB I)
  AntA Q/1 -> Input Buffers/2(AntA Q)
  AntA I/1 -> Input Buffers/1(AntA I)
  Payload/1 -> Delay1/1, Addr_Generator/1(Payload), Symbol Counter/2(Payload), Addr Gen1/2(Payload), Inverter2/1
  Addr_Generator/2(Read Addr) -> Input Buffers/7(Rd Addr)
  Addr_Generator/5(FFT Start) -> FFT/3(Start)
  Input Buffers/2(" Q") -> FFT/2(xn_Q)
  Input Buffers/1(" I") -> FFT/1(xn_I)
  Addr_Generator/1(Write Addr) -> Input Buffers/6(Wr Addr)
  Delay4/1 -> SubCar Index/1
  Delay2/1 -> Vout/1
  Output Buffers/2(" Q") -> Y_Q/1
  Channel Estimation & Pilot Processing/2(H-Q) -> H-Q/1
  Channel Estimation & Pilot Processing/1(H-I) -> H-I/1
  Output Buffers/1(" I") -> Y_I/1
  Channel Estimation & Pilot Processing/5(Est Vout) -> Down Sample/1
  Symbol Counter/1(Sym Index) -> Channel Estimation & Pilot Processing/5(Sym Index), Output Buffers/5(SymIndex)
  FFT/4(Vout) -> Channel Estimation & Pilot Processing/4(Vin), Output Buffers/4(FFT Vout)
  FFT/3(xk_Index) -> Channel Estimation & Pilot Processing/3(Xk Index), Output Buffers/3(Wr Addr)
  FFT/2(xk_Q) -> Output Buffers/2(Q), Channel Estimation & Pilot Processing/2(Q)
  FFT/1(xk_I) -> Channel Estimation & Pilot Processing/1(I), Output Buffers/1(I), [FFT_I]
  FFT/6(xn_Index) -> Assert/1
  Down Sample/1 -> Addr Gen1/3(NotTraining)
  Addr Gen1/2(Rd Addr) -> Output Buffers/6(Rd Addr), Channel Estimation & Pilot Processing/6(Est Index), Delay4/1
  Addr Gen1/3(Vout) -> Delay2/1
  Delay3/1 -> Sym Index/1
  Addr Gen1/1(SymIndex_out) -> Delay3/1
  Addr_Generator/4(Wr Slot) -> Input Buffers/9(Wr Slot)
  Channel Estimation & Pilot Processing/3(Phase I) -> Phase I/1
  Channel Estimation & Pilot Processing/4(Phase Q) -> Phase Q/1
  Inverter2/1 -> Channel Estimation & Pilot Processing/7(Rst)

== system ofdm_txrx_supermimo_coded/OFDM Rx MIMO/Long Corr HP ==
  note: This block was originally designed by Dr. Chris Dick (Cheif DSP Scientist at Xilinx)
      | It's been modified since to run at 4x (instead of 5x, as in the original OFDM designs)
      |
      | This block runs a 64-point 1-bit by 1-bit correlation per clock cycle. Using 1-bit per
      | operand allows just add/sub operations instead of actual multiplicaitons,
      | which would be hugely expensive. This degrades performance a little,
      | but the LTS have good enough autocorrelation properties that there is still
      | plenth of margin using this scheme.
  I In : Inport
  Q In : Inport  Port=2
  AddSub : xbsIndex_r4/AddSub  mode=Subtraction  use_carryin=off  use_carryout=off  en=off  latency=0  precision=Full  arith_type=Unsigned  n_bits=8  bin_pt=2  quantization=Truncate  overflow=Wrap  dbl_ovrd=off  use_behavioral_HDL=on  hw_selection=Fabric  pipelined=off  use_rpm=off  block_type=addsub
      settings: xl_area=[5 0 0 8 0 0 0]  block_version=VER_STRING_GOES_HERE
  AddSub1 : xbsIndex_r4/AddSub  mode=Addition  use_carryin=off  use_carryout=off  en=off  latency=0  precision=Full  arith_type=Unsigned  n_bits=8  bin_pt=2  quantization=Truncate  overflow=Wrap  dbl_ovrd=off  use_behavioral_HDL=on  hw_selection=Fabric  pipelined=off  use_rpm=off  block_type=addsub
      settings: xl_area=[5 0 0 8 0 0 0]  block_version=VER_STRING_GOES_HERE
  AddSub2 : xbsIndex_r4/AddSub  mode=Addition  use_carryin=off  use_carryout=off  en=off  latency=0  precision=Full  arith_type=Unsigned  n_bits=8  bin_pt=2  quantization=Truncate  overflow=Wrap  dbl_ovrd=off  use_behavioral_HDL=on  hw_selection=Fabric  pipelined=off  use_rpm=off  block_type=addsub
      settings: xl_area=[10 0 0 18 0 0 0]  block_version=VER_STRING_GOES_HERE
  C1 : Subsystem  mask{ns=0, N=4, h=longCorr_coef_i, Ts_In=4}
      mask prompts: ns "Num sub-correlator"; N "Coefficients/correlator"; h "Coefficients"; Ts_In "Input sample period"
  C2 : Subsystem  mask{ns=0, N=4, h=longCorr_coef_q, Ts_In=4}
      mask prompts: ns "Num sub-correlator"; N "Coefficients/correlator"; h "Coefficients"; Ts_In "Input sample period"
  C3 : Subsystem  mask{ns=0, N=4, h=longCorr_coef_i, Ts_In=4}
      mask prompts: ns "Num sub-correlator"; N "Coefficients/correlator"; h "Coefficients"; Ts_In "Input sample period"
  C4 : Subsystem  mask{ns=0, N=4, h=longCorr_coef_q, Ts_In=4}
      mask prompts: ns "Num sub-correlator"; N "Coefficients/correlator"; h "Coefficients"; Ts_In "Input sample period"
  Mult : xbsIndex_r4/Mult  precision=Full  arith_type="Signed  (2's comp)"  n_bits=8  bin_pt=2  quantization=Truncate  overflow=Wrap  en=off  latency=1  dbl_ovrd=off  use_behavioral_HDL=off  opt=Speed  use_embedded=on  optimum_pipeline=off  pipeline=on  use_rpm=off  placement_style=Rectangular shape  block_type=mult
      settings: infoedit=Hardware notes: To use the internal pipeline stage of the dedicated multiplier you must select 'Pipeline for maximum performance'.  xl_area=[60 18 0 106 0 0 0]  block_version=VER_STRING_GOES_HERE
  Mult1 : xbsIndex_r4/Mult  precision=Full  arith_type="Signed  (2's comp)"  n_bits=8  bin_pt=2  quantization=Truncate  overflow=Wrap  en=off  latency=1  dbl_ovrd=off  use_behavioral_HDL=off  opt=Speed  use_embedded=on  optimum_pipeline=off  pipeline=on  use_rpm=off  placement_style=Rectangular shape  block_type=mult
      settings: infoedit=Hardware notes: To use the internal pipeline stage of the dedicated multiplier you must select 'Pipeline for maximum performance'.  xl_area=[60 18 0 106 0 0 0]  block_version=VER_STRING_GOES_HERE
  Slice : xbsIndex_r4/Slice  nbits=1  boolean_output=off  mode=Upper Bit Location + Width  bit1=0  base1=MSB of Input  bit0=0  base0=LSB of Input  dbl_ovrd=off  block_type=slice
      settings: infoedit="Extracts a given range of bits from each input sample and presents it at the output.  The output type is ordinarily unsigned with binary point at zero, but can be Boolean when the slice is one bit wide.<P><P>Hardware notes: In hardware this block costs nothing."  block_version=10.1.3
  Slice1 : xbsIndex_r4/Slice  nbits=1  boolean_output=off  mode=Upper Bit Location + Width  bit1=0  base1=MSB of Input  bit0=0  base0=LSB of Input  dbl_ovrd=off  block_type=slice
      settings: infoedit="Extracts a given range of bits from each input sample and presents it at the output.  The output type is ordinarily unsigned with binary point at zero, but can be Boolean when the slice is one bit wide.<P><P>Hardware notes: In hardware this block costs nothing."  block_version=10.1.3
  y : Outport
  tag scope: Long_Corr=global
  wires:
  Slice1/1 -> C2/1(x), C3/1(x)
  Slice/1 -> C1/1(x), C4/1(x)
  C1/1(y) -> AddSub/1
  AddSub2/1 -> y/1, [Long_Corr]
  Mult1/1 -> AddSub2/2
  Mult/1 -> AddSub2/1
  AddSub1/1 -> Mult1/2, Mult1/1
  AddSub/1 -> Mult/2, Mult/1
  C3/1(y) -> AddSub1/2
  C4/1(y) -> AddSub1/1
  C2/1(y) -> AddSub/2
  Q In/1 -> Slice1/1
  I In/1 -> Slice/1

== system ofdm_txrx_supermimo_coded/OFDM Rx MIMO/Packet_Detection ==
  Pkt Active : Inport
  Reset : Inport  Port=2
  Payload : Inport  Port=3
  Auto-Correlation Pkt Det Embedded MULT (for V2P/V6 export) : Subsystem
  Constant : xbsIndex_r4/Constant  const=1  gui_display_data_type=Fixed-point  arith_type=Boolean  n_bits=1  bin_pt=0  preci_type=Single  exp_width=8  frac_width=24  explicit_period=on  period=1  equ=P=C  opselect=C  inp2=PCIN>>17  opr=+  inp1=P  carry=CIN  dbl_ovrd=off  block_type=constant
  Counter1 : xbsIndex_r4/Counter  cnt_type=Free Running  cnt_to=Inf  operation=Up  start_count=0  cnt_by_val=1  arith_type=Unsigned  n_bits=32  bin_pt=0  load_pin=off  rst=off  en=on  explicit_period=off  period=4  dbl_ovrd=off  use_behavioral_HDL=off  implementation=Fabric  use_rpm=off  block_type=counter
  Ext PktDet : Subsystem
  Inverter4 : xbsIndex_r4/Inverter  en=off  latency=0  dbl_ovrd=off  block_type=inv
      settings: block_version=8.2
  Inverter7 : xbsIndex_r4/Inverter  en=off  latency=0  dbl_ovrd=off  block_type=inv
      settings: block_version=8.2
  Logical2 : xbsIndex_r4/Logical  logical_function=OR  inputs=3  en=off  latency=0  precision=Full  arith_type=Unsigned  n_bits=16  bin_pt=0  align_bp=on  dbl_ovrd=off  block_type=logical
      settings: block_version=8.2
  Logical3 : xbsIndex_r4/Logical  logical_function=AND  inputs=2  en=off  latency=0  precision=Full  arith_type=Unsigned  n_bits=16  bin_pt=0  align_bp=on  dbl_ovrd=off  block_type=logical
      settings: block_version=8.2
  Logical4 : xbsIndex_r4/Logical  logical_function=AND  inputs=3  en=off  latency=0  precision=Full  arith_type=Unsigned  n_bits=16  bin_pt=0  align_bp=on  dbl_ovrd=off  block_type=logical
      settings: block_version=8.2
  Logical5 : xbsIndex_r4/Logical  logical_function=OR  inputs=2  en=off  latency=0  precision=Full  arith_type=Unsigned  n_bits=16  bin_pt=0  align_bp=on  dbl_ovrd=off  block_type=logical
      settings: block_version=8.2
  Logical6 : xbsIndex_r4/Logical  logical_function=AND  inputs=2  en=off  latency=0  precision=Full  arith_type=Unsigned  n_bits=16  bin_pt=0  align_bp=on  dbl_ovrd=off  block_type=logical
      settings: block_version=8.2
  Posedge : Subsystem
  RSSI-based PktDet : Subsystem
  Register1 : xbsIndex_r4/Register  init=0  rst=off  en=off  dbl_ovrd=off  block_type=register
  Register2 : xbsIndex_r4/Register  init=0  rst=off  en=off  dbl_ovrd=off  block_type=register
  Up Sample1 : xbsIndex_r4/Up Sample  sample_ratio=4  copy_samples=on  en=off  latency=0  dbl_ovrd=off  block_type=usamp
      settings: infoedit="Up samples input data.  Inserted values  can be zeros or copies of the most recent input sample.<P><P>Hardware notes: No hardware is needed if inserted values are copies of the input sample; otherwise, a mux and single bit flip-flop are used."  block_version=8.2
  debug_pktDetAutoCorr : xbsIndex_r4/Gateway Out  inherit_from_input=off  hdl_port=on  timing_constraint=None  locs_specified=off  LOCs={}  UseAsDAC=off  DACChannel='1'  block_type=gatewayout
      settings: infoedit="Gateway out block.  Converts Xilinx fixed point inputs into ouputs of type Simulink integer, double, or fixed point.<P><P>Hardware notes:  In hardware these blocks become top level output ports or are discarded, depending on how they are configured."  block_version=VER_STRING_GOES_HERE
  debug_pktDetRSSI : xbsIndex_r4/Gateway Out  inherit_from_input=off  hdl_port=on  timing_constraint=None  locs_specified=off  LOCs={}  UseAsDAC=off  DACChannel='1'  block_type=gatewayout
      settings: infoedit="Gateway out block.  Converts Xilinx fixed point inputs into ouputs of type Simulink integer, double, or fixed point.<P><P>Hardware notes:  In hardware these blocks become top level output ports or are discarded, depending on how they are configured."  block_version=VER_STRING_GOES_HERE
  pktdet : xbsIndex_r4/Gateway Out  inherit_from_input=off  hdl_port=on  timing_constraint=None  locs_specified=off  LOCs={}  UseAsDAC=off  DACChannel='1'  block_type=gatewayout
      settings: infoedit="Gateway out block.  Converts Xilinx fixed point inputs into ouputs of type Simulink integer, double, or fixed point.<P><P>Hardware notes:  In hardware these blocks become top level output ports or are discarded, depending on how they are configured."  block_version=VER_STRING_GOES_HERE
  Pkt Det : Outport
  tag scope: pktDet=global, pktDet_corr=global, pktDet_rssi=global, regRx_pktDetEventCount=global, regRx_pktDetEventCount_en=global
  wires:
  [AntA_ADC_I_Filt] -> Auto-Correlation Pkt Det Embedded MULT (for V2P/V6 export)/1(AntA I)
  [AntA_ADC_Q_Filt] -> Auto-Correlation Pkt Det Embedded MULT (for V2P/V6 export)/2(AntA Q)
  Reset/1 -> RSSI-based PktDet/3(PktDet Reset), Inverter7/1, Auto-Correlation Pkt Det Embedded MULT (for V2P/V6 export)/3(PktDet Reset)
  Pkt Active/1 -> Logical5/1
  [Tx_Running] -> Logical5/2
  Posedge/1(Q) -> Counter1/1
  Counter1/1 -> Up Sample1/1
  Constant/1 -> [regRx_pktDetEventCount_en]
  Up Sample1/1 -> [regRx_pktDetEventCount]
  Register1/1 -> debug_pktDetRSSI/1
  Inverter4/1 -> Logical4/2
  Inverter7/1 -> Logical4/3
  [regPktDet_IgnoreDetection] -> Inverter4/1
  Logical2/1 -> Logical4/1
  Payload/1 -> RSSI-based PktDet/1(Payload)
  Logical5/1 -> RSSI-based PktDet/2(Force Busy)
  Ext PktDet/1(Det) -> Logical2/1
  Auto-Correlation Pkt Det Embedded MULT (for V2P/V6 export)/1(PktDet) -> Logical3/2
  [regPktDet_enableAutoCorrDet] -> Logical3/1
  RSSI-based PktDet/1(PktDet) -> Logical6/2
  [regPktDet_enableRSSIdet] -> Logical6/1
  Logical6/1 -> Logical2/3, Register1/1, [pktDet_rssi]
  Logical3/1 -> Logical2/2, Register2/1, [pktDet_corr]
  Logical4/1 -> Pkt Det/1, Posedge/1(D), [pktDet], pktdet/1
  Register2/1 -> debug_pktDetAutoCorr/1
